simulink model "wlan_phy_rx_pmd"  (Simulink 7.8)
settings: Solver=VariableStepDiscrete  SolverMode=SingleTasking  StartTime=0.0  StopTime=rx_sim_time  RelTol=1e-3
PostLoadFcn:
  | wlan_phy_rx_init
InitFcn:
  | wlan_phy_rx_init
StartFcn:
  | wlan_phy_rx_init
values from neighbouring files (symbols this model uses):
  barker_seq20 = 0.95 * circshift(barker_seq20, [0 4]) ./ max(abs(barker_seq20))    [wlan_phy_rx_init.m]
  bit_scrambler_lfsr_bytes = sum(bit_scrambler_lfsr_bytes .* repmat(2.^[0:7], 127, 1),2)    [wlan_phy_rx_init.m]
  deinterleave_ROM = [deinterleave_ROM interleave_11n_64QAM zeros(1, 512-length(interleave_11n_64QAM))]    [wlan_phy_rx_init.m]
  longCorr_coef_i = [3*real(longCorr_coef)]    [wlan_phy_rx_init.m]
  longCorr_coef_q = [3*imag(longCorr_coef)]    [wlan_phy_rx_init.m]
  MAX_CP_LEN = 32    [wlan_phy_rx_init.m]
  MAX_NCBPS = NCBPS_11n_64QAM    (= 312)    [wlan_phy_rx_init.m]
  MAX_NUM_BYTES = 2^16-1    (= 65535)    [wlan_phy_rx_init.m]
  MAX_NUM_SC = 64    [wlan_phy_rx_init.m]
  MAX_NUM_SYMS = 600    [wlan_phy_rx_init.m]
  NCBPS_11a_BPSK = 48    [wlan_phy_rx_init.m]
  NDBPS_11a_BPSK12 = 24    [wlan_phy_rx_init.m]
  PHY_SIGNAL_MIN_LEN = 14    [wlan_phy_rx_init.m]
  preFFT_sampBuff_numSamps = 4*MAX_NUM_SC    (= 256)    [wlan_phy_rx_init.m]
  rx_sim_sig_samp_time = 8    [wlan_phy_rx_init.m]
  scr_ind_rev = zeros(1,128)    [wlan_phy_rx_init.m]
undefined symbols (not defined in the file or in its folder): gcbh, mcs_rom_11ag, mcs_rom_11n, xlBlockLoadCallback, xlGetNormalizedPeriod, xlipgetpartsetting
libraries: xbsIndex_r4 x3862, simulink x1, xbsEDKLib_r4 x1
(part 19 of 23)

== system wlan_phy_rx_pmd/Sync & Antenna Sel/LTS Correlation/Correlator/C4/C6 ==
  x : Inport
  vin : Inport  Port=2
  "  " : Inport  Port=3
  "     " : Inport  Port=4
  ASR : xbsIndex_r4/Addressable Shift Register  infer_latency=off  depth=length(coef2)  initVector=[0]  en=on  dbl_ovrd=off  use_behavioral_HDL=off  Optimization=Resource  use_rpm=off  block_type=addrsr
      settings: infoedit="Delay of configurable length.  Any element in the delay line can be addressed and driven onto the output port.<br><br>Hardware notes: This block is implemented using SRL16s. If Virtex-4 or Spartan-3 device is used, multiple SRLC16s are cascaded together."  xl_area=[1 0 0 2 0 0 0]  block_version=VER_STRING_GOES_HERE
  Delay : xbsIndex_r4/Delay  rst=off  infoeditControl=Selection of Reset will increase slice count due to use of real FFs and instead of SRLs  en=off  latency=1  dbl_ovrd=off  reg_retiming=off  block_type=delay
  Gateway Out{1..8} (8 alike) : xbsIndex_r4/Gateway Out  inherit_from_input=off  hdl_port=off  timing_constraint=None  locs_specified=off  LOCs={}  UseAsDAC=off  DACChannel='1'  block_type=gatewayout
      settings: infoedit="Gateway out block.  Converts Xilinx fixed point inputs into ouputs of type Simulink integer, double, or fixed point.<P><P>Hardware notes:  In hardware these blocks become top level output ports or are discarded, depending on how they are configured."  xl_area=[0 0 0 0 0 0 0]  block_version=VER_STRING_GOES_HERE
  MAC : Subsystem
  ROM : xbsIndex_r4/ROM  depth=length(coef2)  initVector=coef2  distributed_mem=Distributed memory  rst=off  init_reg=0  en=off  latency=1  gui_display_data_type=Fixed-point  arith_type="Signed  (2's comp)"  n_bits=longCorr_coef_nbits  bin_pt=longCorr_coef_bp  preci_type=Single  exp_width=8  frac_width=24  dbl_ovrd=off  optimize=Area  use_rpm=off  block_type=sprom
      settings: xl_area=[1 1 0 1 0 0 0]  block_version=VER_STRING_GOES_HERE
  x_dly : Outport
  x_dly_vout : Outport  Port=2
  y : Outport  Port=3
  y_vout : Outport  Port=4
  wires:
  [ltsCorr_ld] -> MAC/3(ld), Delay/1, Gateway Out3/1
  ROM/1 -> MAC/2(coeff), Gateway Out2/1
  MAC/1(yn) -> y/1, Gateway Out4/1
  ASR/1 -> MAC/1(a), x_dly/1, Gateway Out1/1
  [ltsCorr_cAddr] -> ROM/1, Gateway Out5/1
  [ltsCorr_dAddr] -> ASR/2, Gateway Out7/1
  x/1 -> ASR/1, Gateway Out6/1
  vin/1 -> ASR/3, Gateway Out8/1, MAC/4(rst), x_dly_vout/1
  Delay/1 -> y_vout/1
  Gateway Out8/1 -> ?  "X Vin"
  Gateway Out7/1 -> ?  "ASR Addr"
  Gateway Out6/1 -> ?  "X In"
  Gateway Out1/1 -> ?  "ASR Out"
  Gateway Out2/1 -> ?  "ROM Out"
  Gateway Out3/1 -> ?  "Corr Ld"
  Gateway Out4/1 -> ?  "Corr Out"
  Gateway Out5/1 -> ?  "ROM Addr"

== system wlan_phy_rx_pmd/Sync & Antenna Sel/LTS Correlation/Correlator/C4/C7 ==
  x : Inport
  vin : Inport  Port=2
  "  " : Inport  Port=3
  "     " : Inport  Port=4
  ASR : xbsIndex_r4/Addressable Shift Register  infer_latency=off  depth=length(coef2)  initVector=[0]  en=on  dbl_ovrd=off  use_behavioral_HDL=off  Optimization=Resource  use_rpm=off  block_type=addrsr
      settings: infoedit="Delay of configurable length.  Any element in the delay line can be addressed and driven onto the output port.<br><br>Hardware notes: This block is implemented using SRL16s. If Virtex-4 or Spartan-3 device is used, multiple SRLC16s are cascaded together."  xl_area=[1 0 0 2 0 0 0]  block_version=VER_STRING_GOES_HERE
  Delay : xbsIndex_r4/Delay  rst=off  infoeditControl=Selection of Reset will increase slice count due to use of real FFs and instead of SRLs  en=off  latency=1  dbl_ovrd=off  reg_retiming=off  block_type=delay
  Gateway Out{1..8} (8 alike) : xbsIndex_r4/Gateway Out  inherit_from_input=off  hdl_port=off  timing_constraint=None  locs_specified=off  LOCs={}  UseAsDAC=off  DACChannel='1'  block_type=gatewayout
      settings: infoedit="Gateway out block.  Converts Xilinx fixed point inputs into ouputs of type Simulink integer, double, or fixed point.<P><P>Hardware notes:  In hardware these blocks become top level output ports or are discarded, depending on how they are configured."  xl_area=[0 0 0 0 0 0 0]  block_version=VER_STRING_GOES_HERE
  MAC : Subsystem
  ROM : xbsIndex_r4/ROM  depth=length(coef2)  initVector=coef2  distributed_mem=Distributed memory  rst=off  init_reg=0  en=off  latency=1  gui_display_data_type=Fixed-point  arith_type="Signed  (2's comp)"  n_bits=longCorr_coef_nbits  bin_pt=longCorr_coef_bp  preci_type=Single  exp_width=8  frac_width=24  dbl_ovrd=off  optimize=Area  use_rpm=off  block_type=sprom
      settings: xl_area=[1 1 0 1 0 0 0]  block_version=VER_STRING_GOES_HERE
  x_dly : Outport
  x_dly_vout : Outport  Port=2
  y : Outport  Port=3
  y_vout : Outport  Port=4
  wires:
  Gateway Out5/1 -> ?  "ROM Addr"
  Gateway Out4/1 -> ?  "Corr Out"
  Gateway Out3/1 -> ?  "Corr Ld"
  Gateway Out2/1 -> ?  "ROM Out"
  Gateway Out1/1 -> ?  "ASR Out"
  Gateway Out6/1 -> ?  "X In"
  Gateway Out7/1 -> ?  "ASR Addr"
  Gateway Out8/1 -> ?  "X Vin"
  Delay/1 -> y_vout/1
  vin/1 -> x_dly_vout/1, MAC/4(rst), Gateway Out8/1, ASR/3
  x/1 -> Gateway Out6/1, ASR/1
  [ltsCorr_dAddr] -> Gateway Out7/1, ASR/2
  [ltsCorr_cAddr] -> Gateway Out5/1, ROM/1
  ASR/1 -> Gateway Out1/1, x_dly/1, MAC/1(a)
  MAC/1(yn) -> Gateway Out4/1, y/1
  ROM/1 -> Gateway Out2/1, MAC/2(coeff)
  [ltsCorr_ld] -> Gateway Out3/1, Delay/1, MAC/3(ld)

== system wlan_phy_rx_pmd/Sync & Antenna Sel/LTS Correlation/Correlator/C4/C8 ==
  x : Inport
  vin : Inport  Port=2
  "  " : Inport  Port=3
  "     " : Inport  Port=4
  ASR : xbsIndex_r4/Addressable Shift Register  infer_latency=off  depth=length(coef2)  initVector=[0]  en=on  dbl_ovrd=off  use_behavioral_HDL=off  Optimization=Resource  use_rpm=off  block_type=addrsr
      settings: infoedit="Delay of configurable length.  Any element in the delay line can be addressed and driven onto the output port.<br><br>Hardware notes: This block is implemented using SRL16s. If Virtex-4 or Spartan-3 device is used, multiple SRLC16s are cascaded together."  xl_area=[1 0 0 2 0 0 0]  block_version=VER_STRING_GOES_HERE
  Delay : xbsIndex_r4/Delay  rst=off  infoeditControl=Selection of Reset will increase slice count due to use of real FFs and instead of SRLs  en=off  latency=1  dbl_ovrd=off  reg_retiming=off  block_type=delay
  Gateway Out{1..8} (8 alike) : xbsIndex_r4/Gateway Out  inherit_from_input=off  hdl_port=off  timing_constraint=None  locs_specified=off  LOCs={}  UseAsDAC=off  DACChannel='1'  block_type=gatewayout
      settings: infoedit="Gateway out block.  Converts Xilinx fixed point inputs into ouputs of type Simulink integer, double, or fixed point.<P><P>Hardware notes:  In hardware these blocks become top level output ports or are discarded, depending on how they are configured."  xl_area=[0 0 0 0 0 0 0]  block_version=VER_STRING_GOES_HERE
  MAC : Subsystem
  ROM : xbsIndex_r4/ROM  depth=length(coef2)  initVector=coef2  distributed_mem=Distributed memory  rst=off  init_reg=0  en=off  latency=1  gui_display_data_type=Fixed-point  arith_type="Signed  (2's comp)"  n_bits=longCorr_coef_nbits  bin_pt=longCorr_coef_bp  preci_type=Single  exp_width=8  frac_width=24  dbl_ovrd=off  optimize=Area  use_rpm=off  block_type=sprom
      settings: xl_area=[1 1 0 1 0 0 0]  block_version=VER_STRING_GOES_HERE
  x_dly : Outport
  x_dly_vout : Outport  Port=2
  y : Outport  Port=3
  y_vout : Outport  Port=4
  wires:
  [ltsCorr_ld] -> MAC/3(ld), Delay/1, Gateway Out3/1
  ROM/1 -> MAC/2(coeff), Gateway Out2/1
  MAC/1(yn) -> y/1, Gateway Out4/1
  ASR/1 -> MAC/1(a), x_dly/1, Gateway Out1/1
  [ltsCorr_cAddr] -> ROM/1, Gateway Out5/1
  [ltsCorr_dAddr] -> ASR/2, Gateway Out7/1
  x/1 -> ASR/1, Gateway Out6/1
  vin/1 -> ASR/3, Gateway Out8/1, MAC/4(rst), x_dly_vout/1
  Delay/1 -> y_vout/1
  Gateway Out8/1 -> ?  "X Vin"
  Gateway Out7/1 -> ?  "ASR Addr"
  Gateway Out6/1 -> ?  "X In"
  Gateway Out1/1 -> ?  "ASR Out"
  Gateway Out2/1 -> ?  "ROM Out"
  Gateway Out3/1 -> ?  "Corr Ld"
  Gateway Out4/1 -> ?  "Corr Out"
  Gateway Out5/1 -> ?  "ROM Addr"

== system wlan_phy_rx_pmd/Sync & Antenna Sel/LTS Correlation/Correlator/FSM/S-R Latch ==
  S : Inport
  R : Inport  Port=2
  Constant1 : xbsIndex_r4/Constant  const=1  gui_display_data_type=Fixed-point  arith_type=Boolean  n_bits=1  bin_pt=0  preci_type=Single  exp_width=8  frac_width=24  explicit_period=off  period=1  equ=P=C  opselect=C  inp2=PCIN>>17  opr=+  inp1=P  carry=CIN  dbl_ovrd=off  block_type=constant
      settings: block_version=VER_STRING_GOES_HERE
  Convert1 : xbsIndex_r4/Convert  gui_display_data_type=Boolean  arith_type=Boolean  n_bits=1  bin_pt=0  float_type=Single  exp_bits=8  fraction_bits=24  quantization=Truncate  overflow=Wrap  en=off  latency=0  dbl_ovrd=off  pipeline=off  block_type=convert
  Convert2 : xbsIndex_r4/Convert  gui_display_data_type=Boolean  arith_type=Boolean  n_bits=1  bin_pt=0  float_type=Single  exp_bits=8  fraction_bits=24  quantization=Truncate  overflow=Wrap  en=off  latency=0  dbl_ovrd=off  pipeline=off  block_type=convert
  Register2 : xbsIndex_r4/Register  init=0  rst=on  en=on  dbl_ovrd=off  block_type=register
      settings: xl_area=[1 1 0 0 0 0 0]  block_version=VER_STRING_GOES_HERE
  Q : Outport
  wires:
  Convert2/1 -> Register2/3
  Convert1/1 -> Register2/2
  S/1 -> Convert2/1
  Register2/1 -> Q/1
  R/1 -> Convert1/1
  Constant1/1 -> Register2/1

== system wlan_phy_rx_pmd/Sync & Antenna Sel/PHY CCA/Rate*Length BUSY/Bits/S-R Latch3 ==
  S : Inport
  R : Inport  Port=2
  Constant1 : xbsIndex_r4/Constant  const=1  gui_display_data_type=Fixed-point  arith_type=Boolean  n_bits=1  bin_pt=0  preci_type=Single  exp_width=8  frac_width=24  explicit_period=off  period=1  equ=P=C  opselect=C  inp2=PCIN>>17  opr=+  inp1=P  carry=CIN  dbl_ovrd=off  block_type=constant
      settings: block_version=VER_STRING_GOES_HERE
  Convert1 : xbsIndex_r4/Convert  gui_display_data_type=Boolean  arith_type=Boolean  n_bits=1  bin_pt=0  float_type=Single  exp_bits=8  fraction_bits=24  quantization=Truncate  overflow=Wrap  en=off  latency=0  dbl_ovrd=off  pipeline=off  block_type=convert
  Convert2 : xbsIndex_r4/Convert  gui_display_data_type=Boolean  arith_type=Boolean  n_bits=1  bin_pt=0  float_type=Single  exp_bits=8  fraction_bits=24  quantization=Truncate  overflow=Wrap  en=off  latency=0  dbl_ovrd=off  pipeline=off  block_type=convert
  Register2 : xbsIndex_r4/Register  init=0  rst=on  en=on  dbl_ovrd=off  block_type=register
      settings: xl_area=[1 1 0 0 0 0 0]  block_version=VER_STRING_GOES_HERE
  Q : Outport
  wires:
  Convert2/1 -> Register2/3
  Convert1/1 -> Register2/2
  S/1 -> Convert2/1
  Register2/1 -> Q/1
  R/1 -> Convert1/1
  Constant1/1 -> Register2/1

== system wlan_phy_rx_pmd/Sync & Antenna Sel/PHY CCA/Rate*Length BUSY/Bits/Subsystem ==
  note: This logic resolves a tricky race condition.
      |
      | The PHY does not know whether a given Rx is an 11a or 11n waveform until
      | after the third OFDM symbol following the LTF (SIGNAL + 2 payload
      | symbols). This is fine in most places- the Rx pipeline decodes the
      | SIGNAl and HT-SIG sybmols as quickly as possible, then uses their
      | contents to configure the demod blocks.
      |
      | The problem is some 11a Rx end after the first two payload symbols,
      | typically ACKs at 24Mbps (16QAM 1/2), where the full payload occupies
      | only 2 OFDM symbols after the SIGNAL field. Thus this block cannot wait
      | for the 11a vs 11n decision. The packet might be done by then, and the
      | MAC RX_END must be asserted on schedule for normal operation.
      |
      | This block uses a simple heuristic to resolve this race. For 11n
      | receptions the SIGNAL field always has RATE=6Mbps (BPSK 1/2). An 11a Rx
      | with RATE=6Mbps is also possible, but will always occupy more than 2
      | payload OFDM symbols. Thus this block uses the SIGNAL field RATE
      | exclusively if it is >6Mbps, ignoring the later 11a vs 11n decision
      | supplied via the PARAMS_Valid flag. This allows the rate*length logic to
      | complete in the worst-case (after 2 OFDM symbols for 24Mbps ACKs), while
      | still using the HT-SIG MCS/LENGTH for actual 11n waveforms if present.
  Constant2 : xbsIndex_r4/Constant  const=24  gui_display_data_type=Fixed-point  arith_type=Unsigned  n_bits=5  bin_pt=0  preci_type=Single  exp_width=8  frac_width=24  explicit_period=off  period=1  equ=P=C  opselect=C  inp2=PCIN>>17  opr=+  inp1=P  carry=CIN  dbl_ovrd=off  block_type=constant
  Logical1 : xbsIndex_r4/Logical  logical_function=OR  inputs=2  en=off  latency=0  precision=Full  arith_type=Unsigned  n_bits=16  bin_pt=0  align_bp=on  dbl_ovrd=off  block_type=logical
  Logical2 : xbsIndex_r4/Logical  logical_function=AND  inputs=2  en=off  latency=0  precision=Full  arith_type=Unsigned  n_bits=16  bin_pt=0  align_bp=on  dbl_ovrd=off  block_type=logical
  Posedge : Subsystem
  Register3 : xbsIndex_r4/Register  init=0  rst=off  en=on  dbl_ovrd=off  block_type=register
  Relational1 : xbsIndex_r4/Relational  mode=a=b  en=off  latency=0  dbl_ovrd=off  block_type=relational
  Capt N_DBPS : Outport
  Capt N_SS : Outport  Port=2
  wires:
  Logical1/1 -> Posedge/1(D)
  [OFDM_RX_SIGNAL_VALID] -> Logical1/1, Register3/2
  Logical2/1 -> Logical1/2, Capt N_SS/1
  Constant2/1 -> Relational1/2
  Register3/1 -> Relational1/1
  [OFDM_RX_PARAMS_Valid] -> Logical2/1
  Relational1/1 -> Logical2/2
  [OFDM_RX_DATA_N_DBPS_1] -> Register3/1
  Posedge/1(Q) -> Capt N_DBPS/1

== system wlan_phy_rx_pmd/Sync & Antenna Sel/PHY CCA/Rate*Length BUSY/Extension/S-R Latch1 ==
  S : Inport
  R : Inport  Port=2
  Constant1 : xbsIndex_r4/Constant  const=1  gui_display_data_type=Fixed-point  arith_type=Boolean  n_bits=1  bin_pt=0  preci_type=Single  exp_width=8  frac_width=24  explicit_period=off  period=1  equ=P=C  opselect=C  inp2=PCIN>>17  opr=+  inp1=P  carry=CIN  dbl_ovrd=off  block_type=constant
      settings: block_version=VER_STRING_GOES_HERE
  Convert1 : xbsIndex_r4/Convert  gui_display_data_type=Boolean  arith_type=Boolean  n_bits=1  bin_pt=0  float_type=Single  exp_bits=8  fraction_bits=24  quantization=Truncate  overflow=Wrap  en=off  latency=0  dbl_ovrd=off  pipeline=off  block_type=convert
  Convert2 : xbsIndex_r4/Convert  gui_display_data_type=Boolean  arith_type=Boolean  n_bits=1  bin_pt=0  float_type=Single  exp_bits=8  fraction_bits=24  quantization=Truncate  overflow=Wrap  en=off  latency=0  dbl_ovrd=off  pipeline=off  block_type=convert
  Register2 : xbsIndex_r4/Register  init=0  rst=on  en=on  dbl_ovrd=off  block_type=register
      settings: xl_area=[1 1 0 0 0 0 0]  block_version=VER_STRING_GOES_HERE
  Q : Outport
  wires:
  Constant1/1 -> Register2/1
  R/1 -> Convert1/1
  Register2/1 -> Q/1
  S/1 -> Convert2/1
  Convert1/1 -> Register2/2
  Convert2/1 -> Register2/3

== system wlan_phy_rx_pmd/Sync & Antenna Sel/PHY CCA/Rate*Length BUSY/OFDM Syms/S-R Latch1 ==
  S : Inport
  R : Inport  Port=2
  Constant1 : xbsIndex_r4/Constant  const=1  gui_display_data_type=Fixed-point  arith_type=Boolean  n_bits=1  bin_pt=0  preci_type=Single  exp_width=8  frac_width=24  explicit_period=off  period=1  equ=P=C  opselect=C  inp2=PCIN>>17  opr=+  inp1=P  carry=CIN  dbl_ovrd=off  block_type=constant
      settings: block_version=VER_STRING_GOES_HERE
  Convert1 : xbsIndex_r4/Convert  gui_display_data_type=Boolean  arith_type=Boolean  n_bits=1  bin_pt=0  float_type=Single  exp_bits=8  fraction_bits=24  quantization=Truncate  overflow=Wrap  en=off  latency=0  dbl_ovrd=off  pipeline=off  block_type=convert
  Convert2 : xbsIndex_r4/Convert  gui_display_data_type=Boolean  arith_type=Boolean  n_bits=1  bin_pt=0  float_type=Single  exp_bits=8  fraction_bits=24  quantization=Truncate  overflow=Wrap  en=off  latency=0  dbl_ovrd=off  pipeline=off  block_type=convert
  Register2 : xbsIndex_r4/Register  init=0  rst=on  en=on  dbl_ovrd=off  block_type=register
      settings: xl_area=[1 1 0 0 0 0 0]  block_version=VER_STRING_GOES_HERE
  Q : Outport
  wires:
  Constant1/1 -> Register2/1
  R/1 -> Convert1/1
  Register2/1 -> Q/1
  S/1 -> Convert2/1
  Convert1/1 -> Register2/2
  Convert2/1 -> Register2/3

== system wlan_phy_rx_pmd/Sync & Antenna Sel/Pkt Det/RF A Det/Auto-Corr Pkt Det/Auto Corr ==
  Valid : Inport
  I : Inport  Port=2
  Q : Inport  Port=3
  Avg Mag : Subsystem
  Avg Mag1 : Subsystem
  Conj Mult : Subsystem
  Conj Mult1 : Subsystem
  Delay : xbsIndex_r4/Delay  rst=off  infoeditControl=Selection of Reset will increase slice count due to use of real FFs and instead of SRLs  en=on  latency=16  dbl_ovrd=off  reg_retiming=off  block_type=delay
      settings: xl_area=[7 14 0 14 0 0 0]  block_version=VER_STRING_GOES_HERE
  Delay1 : xbsIndex_r4/Delay  rst=off  infoeditControl=Selection of Reset will increase slice count due to use of real FFs and instead of SRLs  en=on  latency=16  dbl_ovrd=off  reg_retiming=off  block_type=delay
      settings: xl_area=[7 14 0 14 0 0 0]  block_version=VER_STRING_GOES_HERE
  Delay2 : xbsIndex_r4/Delay  rst=off  infoeditControl=Selection of Reset will increase slice count due to use of real FFs and instead of SRLs  en=on  latency=4  dbl_ovrd=off  reg_retiming=off  block_type=delay
      settings: xl_area=[7 14 0 14 0 0 0]  block_version=VER_STRING_GOES_HERE
  Delay3 : xbsIndex_r4/Delay  rst=off  infoeditControl=Selection of Reset will increase slice count due to use of real FFs and instead of SRLs  en=on  latency=4  dbl_ovrd=off  reg_retiming=off  block_type=delay
      settings: xl_area=[7 14 0 14 0 0 0]  block_version=VER_STRING_GOES_HERE
  Register{,1..9} (10 alike) : xbsIndex_r4/Register  init=0  rst=off  en=off  dbl_ovrd=off  block_type=register
  Corr_16 : Outport
  Corr_20 : Outport  Port=2
  wires:
  I/1 -> Delay/1, Register1/1, Register6/1
  Q/1 -> Delay1/1, Register2/1, Register7/1
  Delay/1 -> Register3/1, Delay2/1
  Delay1/1 -> Register4/1, Delay3/1
  Valid/1 -> Delay/2, Delay1/2, Delay2/2, Delay3/2, Register/1, Register5/1
  Conj Mult/2(" I") -> Avg Mag/2(I)
  Conj Mult/3(" Q") -> Avg Mag/3(Q)
  Conj Mult/1(" Valid") -> Avg Mag/1(Valid)
  Avg Mag/1(M) -> Corr_16/1
  Register/1 -> Conj Mult/1(Valid)
  Register1/1 -> Conj Mult/2(A I)
  Register2/1 -> Conj Mult/3(A Q)
  Register3/1 -> Conj Mult/4(B I)
  Register4/1 -> Conj Mult/5(B Q)
  Conj Mult1/2(" I") -> Avg Mag1/2(I)
  Conj Mult1/3(" Q") -> Avg Mag1/3(Q)
  Conj Mult1/1(" Valid") -> Avg Mag1/1(Valid)
  Avg Mag1/1(M) -> Corr_20/1
  Register5/1 -> Conj Mult1/1(Valid)
  Register6/1 -> Conj Mult1/2(A I)
  Register7/1 -> Conj Mult1/3(A Q)
  Delay2/1 -> Register8/1
  Register8/1 -> Conj Mult1/4(B I)
  Register9/1 -> Conj Mult1/5(B Q)
  Delay3/1 -> Register9/1

== system wlan_phy_rx_pmd/Sync & Antenna Sel/Pkt Det/RF A Det/Auto-Corr Pkt Det/Decision ==
  Reset OFDM : Inport
  Reset DSSS : Inport  Port=2
  Corr_16 : Inport  Port=3
  Corr_20 : Inport  Port=4
  Mag : Inport  Port=5
  Auto Corr Decision : Scope  NumInputPorts=8
      settings: TimeRange=45000  YMin=0~0~0~0~-1~-1~-1~-1  YMax=2~4~2~10~1~1~1~1  SaveName=ScopeData43
  Display{,1..7} (8 alike) : Display
  Logical : xbsIndex_r4/Logical  logical_function=AND  inputs=2  en=off  latency=0  precision=Full  arith_type=Unsigned  n_bits=8  bin_pt=2  align_bp=on  dbl_ovrd=off  block_type=logical
      settings: xl_area=[1 0 0 1 0 0 0]  block_version=VER_STRING_GOES_HERE
  Logical1 : xbsIndex_r4/Logical  logical_function=AND  inputs=2  en=off  latency=0  precision=Full  arith_type=Unsigned  n_bits=8  bin_pt=2  align_bp=on  dbl_ovrd=off  block_type=logical
      settings: xl_area=[1 0 0 1 0 0 0]  block_version=VER_STRING_GOES_HERE
  Min Dur : Subsystem
  Min Dur1 : Subsystem
  Mult1 : xbsIndex_r4/Mult  precision=User Defined  arith_type=Unsigned  n_bits=26  bin_pt=14  quantization=Truncate  overflow=Flag as error  en=off  latency=1  dbl_ovrd=off  use_behavioral_HDL=off  opt=Speed  use_embedded=on  optimum_pipeline=off  pipeline=on  use_rpm=off  placement_style=Rectangular shape  block_type=mult
      settings: xl_area=[63 105 0 106 0 4 0]  block_version=VER_STRING_GOES_HERE
  Mult2 : xbsIndex_r4/Mult  precision=User Defined  arith_type=Unsigned  n_bits=26  bin_pt=14  quantization=Truncate  overflow=Flag as error  en=off  latency=1  dbl_ovrd=off  use_behavioral_HDL=off  opt=Speed  use_embedded=on  optimum_pipeline=off  pipeline=on  use_rpm=off  placement_style=Rectangular shape  block_type=mult
      settings: xl_area=[63 105 0 106 0 4 0]  block_version=VER_STRING_GOES_HERE
  Register1 : xbsIndex_r4/Register  init=0  rst=off  en=off  dbl_ovrd=off  block_type=register
  Register2 : xbsIndex_r4/Register  init=0  rst=off  en=off  dbl_ovrd=off  block_type=register
  Register3 : xbsIndex_r4/Register  init=0  rst=off  en=off  dbl_ovrd=off  block_type=register
  Reinterpret{,1..3} (4 alike) : xbsIndex_r4/Reinterpret  force_arith_type=on  arith_type=Unsigned  force_bin_pt=on  bin_pt=0  block_type=reinterpret
  Relational{,1..3} (4 alike) : xbsIndex_r4/Relational  mode=a>b  en=off  latency=1  dbl_ovrd=off  block_type=relational
      settings: xl_area=[11 1 0 22 0 0 0]  block_version=VER_STRING_GOES_HERE
  done{1..12,14..15,17..18} (16 alike) : xbsIndex_r4/Gateway Out  inherit_from_input=off  hdl_port=off  timing_constraint=None  locs_specified=off  LOCs={}  UseAsDAC=off  DACChannel='1'  block_type=gatewayout
      settings: infoedit="Gateway out block.  Converts Xilinx fixed point inputs into ouputs of type Simulink integer, double, or fixed point.<P><P>Hardware notes:  In hardware these blocks become top level output ports or are discarded, depending on how they are configured."  xl_area=[0 0 0 0 1 0 0]  block_version=VER_STRING_GOES_HERE
  Det OFDM : Outport
  Det DSSS : Outport  Port=2
  wires:
  done18/1 -> Display3/1
  Reinterpret1/1 -> done18/1
  done17/1 -> Display2/1
  Reinterpret/1 -> done17/1
  done15/1 -> Display1/1
  done14/1 -> Display/1
  [regRx_PktDet_CorrThresh_A] -> Reinterpret/1, done14/1
  [regRx_PktDet_EnergyThresh_A] -> Reinterpret1/1, done15/1
  Corr_16/1 -> Register3/1
  Mag/1 -> Register1/1
  [regRx_PktDet_CorrThresh_A] -> Mult1/1
  Mult1/1 -> Relational/2, done3/1
  [regRx_PktDet_EnergyThresh_A] -> Relational1/2
  Relational/1 -> Logical/1
  Relational1/1 -> Logical/2
  Relational2/1 -> Logical1/2
  Logical/1 -> done7/1, Min Dur/2("Det ")
  Logical1/1 -> done8/1, Min Dur1/2("Det ")
  [regRx_PktDet_CorrThresh_DSSS_A] -> Mult2/2
  Mult2/1 -> Relational2/2, done6/1
  Corr_20/1 -> Register2/1
  done2/1 -> Display5/1
  Reinterpret2/1 -> done2/1
  done1/1 -> Display4/1
  [regRx_PktDet_CorrThresh_DSSS_A] -> done1/1, Reinterpret2/1
  done4/1 -> Auto Corr Decision/1  "Corr 16"
  done3/1 -> Auto Corr Decision/2  "Scaled Mag 16"
  done5/1 -> Auto Corr Decision/3  "Corr 20"
  done6/1 -> Auto Corr Decision/4  "Scaled Mag 20"
  done7/1 -> Auto Corr Decision/5  "Det 16"
  done8/1 -> Auto Corr Decision/6  "Det 20"
  done9/1 -> Auto Corr Decision/7  "Det OFDM"
  [regRx_PktDet_EnergyThresh_DSSS_A] -> Relational3/2
  Relational3/1 -> Logical1/1
  done11/1 -> Display7/1
  Reinterpret3/1 -> done11/1
  done10/1 -> Display6/1
  [regRx_PktDet_EnergyThresh_DSSS_A] -> done10/1, Reinterpret3/1
  done12/1 -> Auto Corr Decision/8  "Det DSSS"
  Reset OFDM/1 -> Min Dur/1("Reset ")
  Reset DSSS/1 -> Min Dur1/1("Reset ")
  Min Dur/1("Pkt Det ") -> Det OFDM/1, done9/1
  Min Dur1/1("Pkt Det ") -> Det DSSS/1, done12/1
  Register3/1 -> Relational/1, done4/1
  Register1/1 -> Mult1/2, Mult2/1, Relational1/1, Relational3/1
  Register2/1 -> Relational2/1, done5/1

== system wlan_phy_rx_pmd/Sync & Antenna Sel/Pkt Det/RF A Det/Auto-Corr Pkt Det/Mag ==
  Valid : Inport
  I : Inport  Port=2
  Q : Inport  Port=3
  AddSub : xbsIndex_r4/AddSub  mode=Addition  use_carryin=off  use_carryout=off  en=off  latency=0  precision=User Defined  arith_type="Signed  (2's comp)"  n_bits=17  bin_pt=14  quantization=Truncate  overflow=Flag as error  dbl_ovrd=off  use_behavioral_HDL=off  hw_selection=Fabric  pipelined=off  block_type=addsub
  Delay : xbsIndex_r4/Delay  rst=off  infoeditControl=Selection of Reset will increase slice count due to use of real FFs and instead of SRLs  en=off  latency=1  dbl_ovrd=off  reg_retiming=off  block_type=delay
  Mult : xbsIndex_r4/Mult  precision=User Defined  arith_type="Signed  (2's comp)"  n_bits=16  bin_pt=14  quantization=Truncate  overflow=Flag as error  en=off  latency=1  dbl_ovrd=off  use_behavioral_HDL=off  opt=Speed  use_embedded=on  optimum_pipeline=off  pipeline=on  use_rpm=on  placement_style=Triangular  block_type=mult
  Mult1 : xbsIndex_r4/Mult  precision=User Defined  arith_type="Signed  (2's comp)"  n_bits=16  bin_pt=14  quantization=Truncate  overflow=Flag as error  en=off  latency=1  dbl_ovrd=off  use_behavioral_HDL=off  opt=Speed  use_embedded=on  optimum_pipeline=off  pipeline=on  use_rpm=on  placement_style=Triangular  block_type=mult
  Mult2 : xbsIndex_r4/Mult  precision=User Defined  arith_type="Signed  (2's comp)"  n_bits=26  bin_pt=14  quantization=Truncate  overflow=Flag as error  en=on  latency=1  dbl_ovrd=off  use_behavioral_HDL=off  opt=Speed  use_embedded=on  optimum_pipeline=off  pipeline=on  use_rpm=on  placement_style=Triangular  block_type=mult
  Running Sum : Subsystem
  Mag Sq : Outport
  wires:
  Delay/1 -> Running Sum/1(En)
  I/1 -> Mult/1, Mult/2
  Valid/1 -> Delay/1
  Running Sum/1(Sum) -> Mult2/1, Mult2/2
  Q/1 -> Mult1/1, Mult1/2
  Mult/1 -> AddSub/1
  Mult1/1 -> AddSub/2
  AddSub/1 -> Running Sum/2(D)
  Mult2/1 -> Mag Sq/1
  Running Sum/2(Valid) -> Mult2/3

== system wlan_phy_rx_pmd/Sync & Antenna Sel/Pkt Det/RF A Det/RSSI Pkt Det/Detection Decision ==
  note: Require avg(RSSI) > thresh for a minimum number of
      | sample periods. This coutner stops at its max value and
      | is reset any time the avg(RSSI) falls below the threshold.
  Avg RSSI : Inport
  Concat : xbsIndex_r4/Concat  num_inputs=2  dbl_ovrd=off  block_type=concat
  Constant : xbsIndex_r4/Constant  const=0  gui_display_data_type=Fixed-point  arith_type=Unsigned  n_bits=4  bin_pt=0  preci_type=Single  exp_width=8  frac_width=24  explicit_period=off  period=1  equ=P=C  opselect=C  inp2=PCIN>>17  opr=+  inp1=P  carry=CIN  dbl_ovrd=off  block_type=constant
  Counter1 : xbsIndex_r4/Counter  cnt_type=Count Limited  cnt_to=1023  operation=Up  start_count=0  cnt_by_val=1  arith_type=Unsigned  n_bits=10  bin_pt=0  load_pin=off  rst=on  en=on  explicit_period=off  period=4  dbl_ovrd=off  use_behavioral_HDL=off  implementation=Fabric  use_rpm=off  block_type=counter
  Inverter1 : xbsIndex_r4/Inverter  en=off  latency=0  dbl_ovrd=off  block_type=inv
      settings: xl_area=[0 0 0 0 0 0 0]  block_version=8.2
  Register : xbsIndex_r4/Register  init=0  rst=off  en=off  dbl_ovrd=off  block_type=register
  Relational2 : xbsIndex_r4/Relational  mode=a>b  en=off  latency=0  dbl_ovrd=off  block_type=relational
      settings: block_version=VER_STRING_GOES_HERE
  Relational3 : xbsIndex_r4/Relational  mode=a>b  en=off  latency=0  dbl_ovrd=off  block_type=relational
      settings: block_version=VER_STRING_GOES_HERE
  Pkt Det : Outport
  wires:
  [regRx_PKTDET_RSSI_THRESH_A] -> Relational2/2
  Counter1/1 -> Relational3/1
  [regRx_PKTDET_RSSI_MIN_DUR_A] -> Register/1
  Relational3/1 -> Pkt Det/1
  Avg RSSI/1 -> Relational2/1
  Constant/1 -> Concat/2
  Register/1 -> Concat/1
  Concat/1 -> Relational3/2
  Inverter1/1 -> Counter1/1
  Relational2/1 -> Inverter1/1, Counter1/2

== system wlan_phy_rx_pmd/Sync & Antenna Sel/Pkt Det/RF A Det/RSSI Pkt Det/Posedge1 ==
  note: Rising Edge Detection
  D : Inport
  Delay : xbsIndex_r4/Delay  rst=off  infoeditControl=Selection of Reset will increase slice count due to use of real FFs and instead of SRLs  en=off  latency=1  dbl_ovrd=off  reg_retiming=off  block_type=delay
      settings: infoedit=Hardware notes: A delay line is a chain, each link of which is an SRL16 followed by a flip-flop. If register retiming is enabled, the delay line is a chain of flip-flops.  xl_area=[1 1 0 0 0 0 0]  block_version=VER_STRING_GOES_HERE
  Inverter : xbsIndex_r4/Inverter  en=off  latency=0  dbl_ovrd=off  block_type=inv
      settings: xl_area=[0 0 0 0 0 0 0]  block_version=VER_STRING_GOES_HERE
  Logical1 : xbsIndex_r4/Logical  logical_function=AND  inputs=2  en=off  latency=0  precision=Full  arith_type=Unsigned  n_bits=8  bin_pt=2  align_bp=on  dbl_ovrd=off  block_type=logical
      settings: xl_area=[1 0 0 1 0 0 0]  block_version=VER_STRING_GOES_HERE
  Q : Outport
  wires:
  Delay/1 -> Inverter/1
  Inverter/1 -> Logical1/1
  Logical1/1 -> Q/1
  D/1 -> Delay/1, Logical1/2

== system wlan_phy_rx_pmd/Sync & Antenna Sel/Pkt Det/RF A Det/RSSI Pkt Det/S-R Latch1 ==
  S : Inport
  R : Inport  Port=2
  Constant1 : xbsIndex_r4/Constant  const=1  gui_display_data_type=Boolean  arith_type=Boolean  n_bits=1  bin_pt=0  preci_type=Single  exp_width=8  frac_width=24  explicit_period=off  period=1  equ=P=C  opselect=C  inp2=PCIN>>17  opr=+  inp1=P  carry=CIN  dbl_ovrd=off  block_type=constant
      settings: block_version=VER_STRING_GOES_HERE
  Register2 : xbsIndex_r4/Register  init=0  rst=on  en=on  dbl_ovrd=off  block_type=register
      settings: xl_area=[1 1 0 0 0 0 0]  block_version=VER_STRING_GOES_HERE
  Q : Outport
  wires:
  Constant1/1 -> Register2/1
  R/1 -> Register2/2
  Register2/1 -> Q/1
  S/1 -> Register2/3

== system wlan_phy_rx_pmd/Sync & Antenna Sel/Pkt Det/RF A Det/RSSI Pkt Det/S-R Latch2 ==
  S : Inport
  R : Inport  Port=2
  Constant1 : xbsIndex_r4/Constant  const=1  gui_display_data_type=Boolean  arith_type=Boolean  n_bits=1  bin_pt=0  preci_type=Single  exp_width=8  frac_width=24  explicit_period=off  period=1  equ=P=C  opselect=C  inp2=PCIN>>17  opr=+  inp1=P  carry=CIN  dbl_ovrd=off  block_type=constant
      settings: block_version=VER_STRING_GOES_HERE
  Register2 : xbsIndex_r4/Register  init=0  rst=on  en=on  dbl_ovrd=off  block_type=register
      settings: xl_area=[1 1 0 0 0 0 0]  block_version=VER_STRING_GOES_HERE
  Q : Outport
  wires:
  S/1 -> Register2/3
  Register2/1 -> Q/1
  R/1 -> Register2/2
  Constant1/1 -> Register2/1

== system wlan_phy_rx_pmd/Sync & Antenna Sel/Pkt Det/RF B Det/Auto-Corr Pkt Det/Auto Corr ==
  Valid : Inport
  I : Inport  Port=2
  Q : Inport  Port=3
  Avg Mag : Subsystem
  Avg Mag1 : Subsystem
  Conj Mult : Subsystem
  Conj Mult1 : Subsystem
  Delay : xbsIndex_r4/Delay  rst=off  infoeditControl=Selection of Reset will increase slice count due to use of real FFs and instead of SRLs  en=on  latency=16  dbl_ovrd=off  reg_retiming=off  block_type=delay
      settings: xl_area=[7 14 0 14 0 0 0]  block_version=VER_STRING_GOES_HERE
  Delay1 : xbsIndex_r4/Delay  rst=off  infoeditControl=Selection of Reset will increase slice count due to use of real FFs and instead of SRLs  en=on  latency=16  dbl_ovrd=off  reg_retiming=off  block_type=delay
      settings: xl_area=[7 14 0 14 0 0 0]  block_version=VER_STRING_GOES_HERE
  Delay2 : xbsIndex_r4/Delay  rst=off  infoeditControl=Selection of Reset will increase slice count due to use of real FFs and instead of SRLs  en=on  latency=4  dbl_ovrd=off  reg_retiming=off  block_type=delay
      settings: xl_area=[7 14 0 14 0 0 0]  block_version=VER_STRING_GOES_HERE
  Delay3 : xbsIndex_r4/Delay  rst=off  infoeditControl=Selection of Reset will increase slice count due to use of real FFs and instead of SRLs  en=on  latency=4  dbl_ovrd=off  reg_retiming=off  block_type=delay
      settings: xl_area=[7 14 0 14 0 0 0]  block_version=VER_STRING_GOES_HERE
  Register{,1..9} (10 alike) : xbsIndex_r4/Register  init=0  rst=off  en=off  dbl_ovrd=off  block_type=register
  Corr_16 : Outport
  Corr_20 : Outport  Port=2
  wires:
  Delay3/1 -> Register9/1
  Register9/1 -> Conj Mult1/5(B Q)
  Register8/1 -> Conj Mult1/4(B I)
  Delay2/1 -> Register8/1
  Register7/1 -> Conj Mult1/3(A Q)
  Register6/1 -> Conj Mult1/2(A I)
  Register5/1 -> Conj Mult1/1(Valid)
  Avg Mag1/1(M) -> Corr_20/1
  Conj Mult1/1(" Valid") -> Avg Mag1/1(Valid)
  Conj Mult1/3(" Q") -> Avg Mag1/3(Q)
  Conj Mult1/2(" I") -> Avg Mag1/2(I)
  Register4/1 -> Conj Mult/5(B Q)
  Register3/1 -> Conj Mult/4(B I)
  Register2/1 -> Conj Mult/3(A Q)
  Register1/1 -> Conj Mult/2(A I)
  Register/1 -> Conj Mult/1(Valid)
  Avg Mag/1(M) -> Corr_16/1
  Conj Mult/1(" Valid") -> Avg Mag/1(Valid)
  Conj Mult/3(" Q") -> Avg Mag/3(Q)
  Conj Mult/2(" I") -> Avg Mag/2(I)
  Valid/1 -> Register5/1, Register/1, Delay3/2, Delay2/2, Delay1/2, Delay/2
  Delay1/1 -> Delay3/1, Register4/1
  Delay/1 -> Delay2/1, Register3/1
  Q/1 -> Register7/1, Register2/1, Delay1/1
  I/1 -> Register6/1, Register1/1, Delay/1

== system wlan_phy_rx_pmd/Sync & Antenna Sel/Pkt Det/RF B Det/Auto-Corr Pkt Det/Decision ==
  Reset OFDM : Inport
  Reset DSSS : Inport  Port=2
  Corr_16 : Inport  Port=3
  Corr_20 : Inport  Port=4
  Mag : Inport  Port=5
  Auto Corr Decision : Scope  NumInputPorts=8
      settings: TimeRange=10000  YMin=0~0~0~0~-1~-1~0.95~-5  YMax=600~500~60~700~1~1~1.05~5  SaveName=ScopeData43
  Logical : xbsIndex_r4/Logical  logical_function=AND  inputs=2  en=off  latency=0  precision=Full  arith_type=Unsigned  n_bits=8  bin_pt=2  align_bp=on  dbl_ovrd=off  block_type=logical
      settings: xl_area=[1 0 0 1 0 0 0]  block_version=VER_STRING_GOES_HERE
  Logical1 : xbsIndex_r4/Logical  logical_function=AND  inputs=2  en=off  latency=0  precision=Full  arith_type=Unsigned  n_bits=8  bin_pt=2  align_bp=on  dbl_ovrd=off  block_type=logical
      settings: xl_area=[1 0 0 1 0 0 0]  block_version=VER_STRING_GOES_HERE
  Min Dur : Subsystem
  Min Dur1 : Subsystem
  Mult1 : xbsIndex_r4/Mult  precision=User Defined  arith_type=Unsigned  n_bits=26  bin_pt=14  quantization=Truncate  overflow=Flag as error  en=off  latency=1  dbl_ovrd=off  use_behavioral_HDL=off  opt=Speed  use_embedded=on  optimum_pipeline=off  pipeline=on  use_rpm=off  placement_style=Rectangular shape  block_type=mult
      settings: xl_area=[63 105 0 106 0 4 0]  block_version=VER_STRING_GOES_HERE
  Mult2 : xbsIndex_r4/Mult  precision=User Defined  arith_type=Unsigned  n_bits=26  bin_pt=14  quantization=Truncate  overflow=Flag as error  en=off  latency=1  dbl_ovrd=off  use_behavioral_HDL=off  opt=Speed  use_embedded=on  optimum_pipeline=off  pipeline=on  use_rpm=off  placement_style=Rectangular shape  block_type=mult
      settings: xl_area=[63 105 0 106 0 4 0]  block_version=VER_STRING_GOES_HERE
  Register1 : xbsIndex_r4/Register  init=0  rst=off  en=off  dbl_ovrd=off  block_type=register
  Register2 : xbsIndex_r4/Register  init=0  rst=off  en=off  dbl_ovrd=off  block_type=register
  Register3 : xbsIndex_r4/Register  init=0  rst=off  en=off  dbl_ovrd=off  block_type=register
  Relational{,1..3} (4 alike) : xbsIndex_r4/Relational  mode=a>b  en=off  latency=1  dbl_ovrd=off  block_type=relational
      settings: xl_area=[11 1 0 22 0 0 0]  block_version=VER_STRING_GOES_HERE
  done{3..9,12} (8 alike) : xbsIndex_r4/Gateway Out  inherit_from_input=off  hdl_port=off  timing_constraint=None  locs_specified=off  LOCs={}  UseAsDAC=off  DACChannel='1'  block_type=gatewayout
      settings: infoedit="Gateway out block.  Converts Xilinx fixed point inputs into ouputs of type Simulink integer, double, or fixed point.<P><P>Hardware notes:  In hardware these blocks become top level output ports or are discarded, depending on how they are configured."  xl_area=[0 0 0 0 1 0 0]  block_version=VER_STRING_GOES_HERE
  Det OFDM : Outport
  Det DSSS : Outport  Port=2
  wires:
  Register2/1 -> done5/1, Relational2/1
  Register1/1 -> Relational3/1, Relational1/1, Mult2/1, Mult1/2
  Register3/1 -> done4/1, Relational/1
  Min Dur1/1("Pkt Det ") -> done12/1, Det DSSS/1
  Min Dur/1("Pkt Det ") -> done9/1, Det OFDM/1
  Reset DSSS/1 -> Min Dur1/1("Reset ")
  Reset OFDM/1 -> Min Dur/1("Reset ")
  done12/1 -> Auto Corr Decision/8  "Det DSSS"
  Relational3/1 -> Logical1/1
  [regRx_PktDet_EnergyThresh_DSSS_B] -> Relational3/2
  done9/1 -> Auto Corr Decision/7  "Det OFDM"
  done8/1 -> Auto Corr Decision/6  "Det 20"
  done7/1 -> Auto Corr Decision/5  "Det 16"
  done6/1 -> Auto Corr Decision/4  "Scaled Mag 20"
  done5/1 -> Auto Corr Decision/3  "Corr 20"
  done3/1 -> Auto Corr Decision/2  "Scaled Mag 16"
  done4/1 -> Auto Corr Decision/1  "Corr 16"
  Corr_20/1 -> Register2/1
  Mult2/1 -> done6/1, Relational2/2
  [regRx_PktDet_CorrThresh_DSSS_B] -> Mult2/2
  Logical1/1 -> Min Dur1/2("Det "), done8/1
  Logical/1 -> Min Dur/2("Det "), done7/1
  Relational2/1 -> Logical1/2
  Relational1/1 -> Logical/2
  Relational/1 -> Logical/1
  [regRx_PktDet_EnergyThresh_B] -> Relational1/2
  Mult1/1 -> done3/1, Relational/2
  [regRx_PktDet_CorrThresh_B] -> Mult1/1
  Mag/1 -> Register1/1
  Corr_16/1 -> Register3/1

== system wlan_phy_rx_pmd/Sync & Antenna Sel/Pkt Det/RF B Det/Auto-Corr Pkt Det/Mag ==
  Valid : Inport
  I : Inport  Port=2
  Q : Inport  Port=3
  AddSub : xbsIndex_r4/AddSub  mode=Addition  use_carryin=off  use_carryout=off  en=off  latency=0  precision=User Defined  arith_type="Signed  (2's comp)"  n_bits=17  bin_pt=14  quantization=Truncate  overflow=Flag as error  dbl_ovrd=off  use_behavioral_HDL=off  hw_selection=Fabric  pipelined=off  block_type=addsub
  Delay : xbsIndex_r4/Delay  rst=off  infoeditControl=Selection of Reset will increase slice count due to use of real FFs and instead of SRLs  en=off  latency=1  dbl_ovrd=off  reg_retiming=off  block_type=delay
  Mult : xbsIndex_r4/Mult  precision=User Defined  arith_type="Signed  (2's comp)"  n_bits=16  bin_pt=14  quantization=Truncate  overflow=Flag as error  en=off  latency=1  dbl_ovrd=off  use_behavioral_HDL=off  opt=Speed  use_embedded=on  optimum_pipeline=off  pipeline=on  use_rpm=on  placement_style=Triangular  block_type=mult
  Mult1 : xbsIndex_r4/Mult  precision=User Defined  arith_type="Signed  (2's comp)"  n_bits=16  bin_pt=14  quantization=Truncate  overflow=Flag as error  en=off  latency=1  dbl_ovrd=off  use_behavioral_HDL=off  opt=Speed  use_embedded=on  optimum_pipeline=off  pipeline=on  use_rpm=on  placement_style=Triangular  block_type=mult
  Mult2 : xbsIndex_r4/Mult  precision=User Defined  arith_type="Signed  (2's comp)"  n_bits=26  bin_pt=14  quantization=Truncate  overflow=Flag as error  en=on  latency=1  dbl_ovrd=off  use_behavioral_HDL=off  opt=Speed  use_embedded=on  optimum_pipeline=off  pipeline=on  use_rpm=on  placement_style=Triangular  block_type=mult
  Running Sum : Subsystem
  Mag Sq : Outport
  wires:
  Running Sum/2(Valid) -> Mult2/3
  Mult2/1 -> Mag Sq/1
  AddSub/1 -> Running Sum/2(D)
  Mult1/1 -> AddSub/2
  Mult/1 -> AddSub/1
  Q/1 -> Mult1/2, Mult1/1
  Running Sum/1(Sum) -> Mult2/2, Mult2/1
  Valid/1 -> Delay/1
  I/1 -> Mult/2, Mult/1
  Delay/1 -> Running Sum/1(En)

== system wlan_phy_rx_pmd/Sync & Antenna Sel/Pkt Det/RF B Det/RSSI Pkt Det/Detection Decision ==
  note: Require avg(RSSI) > thresh for a minimum number of
      | sample periods. This coutner stops at its max value and
      | is reset any time the avg(RSSI) falls below the threshold.
  Avg RSSI : Inport
  Concat : xbsIndex_r4/Concat  num_inputs=2  dbl_ovrd=off  block_type=concat
  Constant : xbsIndex_r4/Constant  const=0  gui_display_data_type=Fixed-point  arith_type=Unsigned  n_bits=4  bin_pt=0  preci_type=Single  exp_width=8  frac_width=24  explicit_period=off  period=1  equ=P=C  opselect=C  inp2=PCIN>>17  opr=+  inp1=P  carry=CIN  dbl_ovrd=off  block_type=constant
  Counter1 : xbsIndex_r4/Counter  cnt_type=Count Limited  cnt_to=1023  operation=Up  start_count=0  cnt_by_val=1  arith_type=Unsigned  n_bits=10  bin_pt=0  load_pin=off  rst=on  en=on  explicit_period=off  period=4  dbl_ovrd=off  use_behavioral_HDL=off  implementation=Fabric  use_rpm=off  block_type=counter
  Inverter1 : xbsIndex_r4/Inverter  en=off  latency=0  dbl_ovrd=off  block_type=inv
      settings: xl_area=[0 0 0 0 0 0 0]  block_version=8.2
  Register : xbsIndex_r4/Register  init=0  rst=off  en=off  dbl_ovrd=off  block_type=register
  Relational2 : xbsIndex_r4/Relational  mode=a>b  en=off  latency=0  dbl_ovrd=off  block_type=relational
      settings: block_version=VER_STRING_GOES_HERE
  Relational3 : xbsIndex_r4/Relational  mode=a>b  en=off  latency=0  dbl_ovrd=off  block_type=relational
      settings: block_version=VER_STRING_GOES_HERE
  Pkt Det : Outport
  wires:
  Relational2/1 -> Counter1/2, Inverter1/1
  Inverter1/1 -> Counter1/1
  Concat/1 -> Relational3/2
  Register/1 -> Concat/1
  Constant/1 -> Concat/2
  Avg RSSI/1 -> Relational2/1
  Relational3/1 -> Pkt Det/1
  [regRx_PKTDET_RSSI_MIN_DUR_B] -> Register/1
  Counter1/1 -> Relational3/1
  [regRx_PKTDET_RSSI_THRESH_B] -> Relational2/2

== system wlan_phy_rx_pmd/Sync & Antenna Sel/Pkt Det/RF B Det/RSSI Pkt Det/Posedge1 ==
  note: Rising Edge Detection
  D : Inport
  Delay : xbsIndex_r4/Delay  rst=off  infoeditControl=Selection of Reset will increase slice count due to use of real FFs and instead of SRLs  en=off  latency=1  dbl_ovrd=off  reg_retiming=off  block_type=delay
      settings: infoedit=Hardware notes: A delay line is a chain, each link of which is an SRL16 followed by a flip-flop. If register retiming is enabled, the delay line is a chain of flip-flops.  xl_area=[1 1 0 0 0 0 0]  block_version=VER_STRING_GOES_HERE
  Inverter : xbsIndex_r4/Inverter  en=off  latency=0  dbl_ovrd=off  block_type=inv
      settings: xl_area=[0 0 0 0 0 0 0]  block_version=VER_STRING_GOES_HERE
  Logical1 : xbsIndex_r4/Logical  logical_function=AND  inputs=2  en=off  latency=0  precision=Full  arith_type=Unsigned  n_bits=8  bin_pt=2  align_bp=on  dbl_ovrd=off  block_type=logical
      settings: xl_area=[1 0 0 1 0 0 0]  block_version=VER_STRING_GOES_HERE
  Q : Outport
  wires:
  D/1 -> Logical1/2, Delay/1
  Logical1/1 -> Q/1
  Inverter/1 -> Logical1/1
  Delay/1 -> Inverter/1

== system wlan_phy_rx_pmd/Sync & Antenna Sel/Pkt Det/RF B Det/RSSI Pkt Det/S-R Latch1 ==
  S : Inport
  R : Inport  Port=2
  Constant1 : xbsIndex_r4/Constant  const=1  gui_display_data_type=Boolean  arith_type=Boolean  n_bits=1  bin_pt=0  preci_type=Single  exp_width=8  frac_width=24  explicit_period=off  period=1  equ=P=C  opselect=C  inp2=PCIN>>17  opr=+  inp1=P  carry=CIN  dbl_ovrd=off  block_type=constant
      settings: block_version=VER_STRING_GOES_HERE
  Register2 : xbsIndex_r4/Register  init=0  rst=on  en=on  dbl_ovrd=off  block_type=register
      settings: xl_area=[1 1 0 0 0 0 0]  block_version=VER_STRING_GOES_HERE
  Q : Outport
  wires:
  S/1 -> Register2/3
  Register2/1 -> Q/1
  R/1 -> Register2/2
  Constant1/1 -> Register2/1

== system wlan_phy_rx_pmd/Sync & Antenna Sel/Pkt Det/RF B Det/RSSI Pkt Det/S-R Latch2 ==
  S : Inport
  R : Inport  Port=2
  Constant1 : xbsIndex_r4/Constant  const=1  gui_display_data_type=Boolean  arith_type=Boolean  n_bits=1  bin_pt=0  preci_type=Single  exp_width=8  frac_width=24  explicit_period=off  period=1  equ=P=C  opselect=C  inp2=PCIN>>17  opr=+  inp1=P  carry=CIN  dbl_ovrd=off  block_type=constant
      settings: block_version=VER_STRING_GOES_HERE
  Register2 : xbsIndex_r4/Register  init=0  rst=on  en=on  dbl_ovrd=off  block_type=register
      settings: xl_area=[1 1 0 0 0 0 0]  block_version=VER_STRING_GOES_HERE
  Q : Outport
  wires:
  Constant1/1 -> Register2/1
  R/1 -> Register2/2
  Register2/1 -> Q/1
  S/1 -> Register2/3

== system wlan_phy_rx_pmd/Detect & Decode/Decode/SIGNAL & HT-SIG Decode/HT-SIG Decode/CRC8 Calc/Endian Swap ==
  B : Inport
  Concat : xbsIndex_r4/Concat  num_inputs=8  dbl_ovrd=off  block_type=concat
  b0 : xbsIndex_r4/Slice  nbits=1  boolean_output=off  mode=Lower Bit Location + Width  bit1=0  base1=MSB of Input  bit0=0  base0=LSB of Input  dbl_ovrd=off  block_type=slice
  b1 : xbsIndex_r4/Slice  nbits=1  boolean_output=off  mode=Lower Bit Location + Width  bit1=0  base1=MSB of Input  bit0=1  base0=LSB of Input  dbl_ovrd=off  block_type=slice
  b2 : xbsIndex_r4/Slice  nbits=1  boolean_output=off  mode=Lower Bit Location + Width  bit1=0  base1=MSB of Input  bit0=2  base0=LSB of Input  dbl_ovrd=off  block_type=slice
  b3 : xbsIndex_r4/Slice  nbits=1  boolean_output=off  mode=Lower Bit Location + Width  bit1=0  base1=MSB of Input  bit0=3  base0=LSB of Input  dbl_ovrd=off  block_type=slice
  b4 : xbsIndex_r4/Slice  nbits=1  boolean_output=off  mode=Lower Bit Location + Width  bit1=0  base1=MSB of Input  bit0=4  base0=LSB of Input  dbl_ovrd=off  block_type=slice
  b5 : xbsIndex_r4/Slice  nbits=1  boolean_output=off  mode=Lower Bit Location + Width  bit1=0  base1=MSB of Input  bit0=5  base0=LSB of Input  dbl_ovrd=off  block_type=slice
  b6 : xbsIndex_r4/Slice  nbits=1  boolean_output=off  mode=Lower Bit Location + Width  bit1=0  base1=MSB of Input  bit0=6  base0=LSB of Input  dbl_ovrd=off  block_type=slice
  b7 : xbsIndex_r4/Slice  nbits=1  boolean_output=off  mode=Lower Bit Location + Width  bit1=0  base1=MSB of Input  bit0=7  base0=LSB of Input  dbl_ovrd=off  block_type=slice
  I : Outport
  wires:
  B/1 -> b0/1, b1/1, b2/1, b3/1, b4/1, b5/1, b6/1, b7/1
  b0/1 -> Concat/1
  b1/1 -> Concat/2
  b2/1 -> Concat/3
  b3/1 -> Concat/4
  b4/1 -> Concat/5
  b5/1 -> Concat/6
  b6/1 -> Concat/7
  Concat/1 -> I/1
  b7/1 -> Concat/8

== system wlan_phy_rx_pmd/Detect & Decode/Decode/SIGNAL & HT-SIG Decode/SIGNAL Decode/4b Shift Reg/Posedge3 ==
  note: Rising Edge Detection
  D : Inport
  Delay : xbsIndex_r4/Delay  rst=off  infoeditControl=Selection of Reset will increase slice count due to use of real FFs and instead of SRLs  en=off  latency=1  dbl_ovrd=off  reg_retiming=off  block_type=delay
      settings: infoedit=Hardware notes: A delay line is a chain, each link of which is an SRL16 followed by a flip-flop. If register retiming is enabled, the delay line is a chain of flip-flops.  xl_area=[1 1 0 0 0 0 0]  block_version=VER_STRING_GOES_HERE
  Inverter : xbsIndex_r4/Inverter  en=off  latency=0  dbl_ovrd=off  block_type=inv
      settings: xl_area=[0 0 0 0 0 0 0]  block_version=VER_STRING_GOES_HERE
  Logical1 : xbsIndex_r4/Logical  logical_function=AND  inputs=2  en=off  latency=0  precision=Full  arith_type=Unsigned  n_bits=8  bin_pt=2  align_bp=on  dbl_ovrd=off  block_type=logical
      settings: xl_area=[1 0 0 1 0 0 0]  block_version=VER_STRING_GOES_HERE
  Q : Outport
  wires:
  Delay/1 -> Inverter/1
  Inverter/1 -> Logical1/1
  Logical1/1 -> Q/1
  D/1 -> Delay/1, Logical1/2

== system wlan_phy_rx_pmd/Detect & Decode/Decode/SIGNAL & HT-SIG Decode/SIGNAL Decode/Tail & Parity Check/2b XOR ==
  A : Inport
  Logical : xbsIndex_r4/Logical  logical_function=XOR  inputs=2  en=off  latency=0  precision=Full  arith_type=Unsigned  n_bits=16  bin_pt=0  align_bp=on  dbl_ovrd=off  block_type=logical
  b0 : xbsIndex_r4/Slice  nbits=1  boolean_output=off  mode=Lower Bit Location + Width  bit1=0  base1=MSB of Input  bit0=0  base0=LSB of Input  dbl_ovrd=off  block_type=slice
  b1 : xbsIndex_r4/Slice  nbits=1  boolean_output=off  mode=Lower Bit Location + Width  bit1=0  base1=MSB of Input  bit0=1  base0=LSB of Input  dbl_ovrd=off  block_type=slice
  B : Outport
  wires:
  Logical/1 -> B/1
  b1/1 -> Logical/2
  b0/1 -> Logical/1
  A/1 -> b1/1, b0/1
